simulink model "v01_aa_rii_16r4t11f_ver122sa"  (Simulink 7.6)
settings: Solver=ode45  StartTime=0.0  StopTime=128000  RelTol=1e-3
undefined symbols (not defined in the file or in its folder): sfp
libraries: xbsIndex_r4 x27287, monroe_library x154, xbsReplacements_r4 x78, casper_library_misc x33, simulink x32, dspsigops x32, xps_library x24
(part 23 of 178)

== system v01_aa_rii_16r4t11f_ver122sa/pfb_fir_real2/coeff_gen_3_12 ==
  sync : Inport
  Counter0 : xbsIndex_r4/Counter  cnt_type=Free Running  cnt_to=Inf  operation=Up  start_count=8  cnt_by_val=1  arith_type=Unsigned  n_bits=7  bin_pt=0  load_pin=off  rst=on  en=off  explicit_period=on  period=1  dbl_ovrd=off  use_behavioral_HDL=off  implementation=Fabric  block_type=counter
  Counter1 : xbsIndex_r4/Counter  cnt_type=Free Running  cnt_to=Inf  operation=Down  start_count=119  cnt_by_val=1  arith_type=Unsigned  n_bits=7  bin_pt=0  load_pin=off  rst=on  en=off  explicit_period=on  period=1  dbl_ovrd=off  use_behavioral_HDL=off  implementation=Fabric  block_type=counter
  Delay16 : xbsIndex_r4/Delay  rst=off  infoeditControl=Selection of Reset will increase slice count due to use of real FFs and instead of SRLs  en=off  latency=2  dbl_ovrd=off  reg_retiming=off  block_type=delay
  Delay17 : xbsIndex_r4/Delay  rst=off  infoeditControl=Selection of Reset will increase slice count due to use of real FFs and instead of SRLs  en=off  latency=1  dbl_ovrd=off  reg_retiming=off  block_type=delay
  Delay18 : xbsIndex_r4/Delay  rst=off  infoeditControl=Selection of Reset will increase slice count due to use of real FFs and instead of SRLs  en=off  latency=1  dbl_ovrd=off  reg_retiming=off  block_type=delay
  Delay19 : xbsIndex_r4/Delay  rst=off  infoeditControl=Selection of Reset will increase slice count due to use of real FFs and instead of SRLs  en=off  latency=2  dbl_ovrd=off  reg_retiming=off  block_type=delay
  coeff_ram_1 : xbsIndex_r4/Dual Port RAM  depth=128  initVector=[-6.84185504899742e-05 -0.000382857821943778 -0.000700524248323099 -0.00102208509462138 -0.00134821437640948 -0.00167959124907416 -0.00201689836785779 -0.00236082022082727 -0.00271204143695053 -0.00307124507150638 -0.00343911087109885 -0.00381631352058928 -0.00420352087429949 -0.00460139217387592 -0.00501057625523885 -0.00543170974707191 -0.0058654152633352 -0.00631229959231075 -0.00677295188471086 -0.00724794184339896 -0.00773781791728902 -0.00824310550200131 -0.00876430514986291 -0.00930189079184692 -0.00985630797404788 -0.0104279721112905 -0.0110172667604652 -0.0116245419161785 -0.0122501123312931 -0.0128942558649243 -0.0135572118604367 -0.0142391795559714 -0.0149403165300052 -0.0156607371844211 -0.0164005112675375 -0.0171596624395113 -0.0179381668824935 -0.0187359519578779 -0.0195528949129399 -0.0203888216391169 -0.0212435054841348 -0.0221166661201311 -0.0230079684698733 -0.0239170216931123 -0.0248433782350494 -0.0257865329388359 -0.0267459222239541 -0.0277209233322634 -0.0287108536434236 -0.029714970061332 -0.0307324684731381 -0.0317624832823181 -0.0328040870172151 -0.0338562900163623 -0.0349180401918279 -0.0359882228717288 -0.0370656607229749 -0.038149113755212 -0.0392372794068422 -0.0403287927139051 -0.0414222265625081 -0.0425160920253971 -0.0436088387831623 -0.0446988556304727 -0.0457844710676339 -0.0468639539776635 -0.0479355143889729 -0.0489973043236477 -0.050047418731209 -0.0510838965076389 -0.0521047215993477 -0.0531078241916569 -0.0540910819812655 -0.0550523215320667 -0.0559893197135738 -0.0568998052211139 -0.0577814601768411 -0.0586319218105215 -0.059448784218937 -0.0602296002026545 -0.0609718831788084 -0.0616731091684402 -0.0623307188568461 -0.0629421197252817 -0.0635046882522795 -0.0640157721827404 -0.0644726928628674 -0.0648727476389184 -0.0652132123176663 -0.0654913436863688 -0.0657043820899625 -0.0658495540631149 -0.0659240750146882 -0.0659251519620883 -0.0658499863128975 -0.0656957766911188 -0.0654597218052851 -0.0651390233556215 -0.0647308889773858 -0.0642325352174459 -0.0636411905410978 -0.0629540983660725 -0.0621685201206242 -0.0612817383225471 -0.0602910596759198 -0.0591938181823348 -0.0579873782633317 -0.0566691378907179 -0.055236531721427 -0.0536870342335396 -0.0520181628600644 -0.0502274811170587 -0.0483126017226495 -0.0462711897035023 -0.0441009654852791 -0.0417997079636163 -0.0393652575521568 -0.0367955192041694 -0.0340884654042976 -0.0312421391269863 -0.028254656758155 -0.0251242109766973 -0.021849073592414 -0.0184275983370102 -0.0148582236048162 -0.0111394751399288 -0.00726996866650266 -0.00324841245896634]  distributed_mem=Block RAM  init_a=0  init_b=0  rst_a=off  rst_b=off  en_a=off  en_b=off  latency=2  write_mode_A=No Read On Write  write_mode_B=No Read On Write  dbl_ovrd=off  optimize=Area  block_type=dpram
  coeff_ram_2 : xbsIndex_r4/Dual Port RAM  depth=128  initVector=[0.000926390151018473 0.00525553833398869 0.00974003139158092 0.0143807664579271 0.0191785362765084 0.0241340270553677 0.029247816403515 0.0345203713512892 0.0399520464573652 0.0455430820050192 0.0512936022901863 0.0572036140037557 0.0632730047104714 0.0695015414267057 0.0758888692992913 0.0824345103874953 0.0891378625501222 0.0959981984396374 0.103014664605091 0.110186280705523 0.117511938835429 0.124990402963731 0.132620308487624 0.140400161902524 0.148328340589239 0.156403092719369 0.164622537279825 0.172984664217222 0.181487334702801 0.190128281518395 0.198905109563833 0.20781529648607 0.216856193430172 0.226025025912192 0.235318894813825 0.244734777498616 0.254269529049357 0.263919883626188 0.273682455944801 0.283553742873988 0.293530125151685 0.303607869218511 0.313783129167691 0.324051948810107 0.334410263853137 0.344853904191773 0.355378596310409 0.36597996579359 0.376653539943846 0.387394750504671 0.398198936486543 0.409061347093818 0.419977144750163 0.430941408220135 0.441949135824379 0.452995248745804 0.464074594424031 0.475181950035267 0.486312026054677 0.497459469898254 0.508618869641048 0.519784757808577 0.530951615238117 0.54211387500652 0.553265926421101 0.564402119070086 0.575516766929012 0.586604152519439 0.597658531116231 0.608674134999635 0.619645177748309 0.6305658585694 0.641430366661742 0.652232885608173 0.662967597792947 0.673628688840178 0.684210352069207 0.694706792962786 0.705112233643903 0.7154209173571 0.725627112950088 0.735725119351461 0.745709270040315 0.755573937503563 0.765313537676731 0.774922534364069 0.784395443633751 0.793726838184032 0.802911351676187 0.811943683030113 0.820818600678503 0.829530946775514 0.838075641355911 0.846447686440694 0.854642170085277 0.862654270366307 0.870479259303306 0.87811250671135 0.885549483981054 0.892785767782227 0.899817043687609 0.906639109713177 0.913247879771583 0.919639387035394 0.925809787206842 0.931755361690913 0.937472520668701 0.942957806068012 0.948207894428338 0.953219599657401 0.957989875676573 0.962515818952598 0.966794670913127 0.970823820243721 0.974600805064058 0.978123314981223 0.981389193018064 0.984396437414738 0.98714320330165 0.989627804242188 0.991848713643696 0.993804566035335 0.995494158211552 0.996916450240055 0.998070566333287 0.998955795582552 0.999571592554069 0.999917577746371]  distributed_mem=Block RAM  init_a=0  init_b=0  rst_a=off  rst_b=off  en_a=off  en_b=off  latency=2  write_mode_A=No Read On Write  write_mode_B=No Read On Write  dbl_ovrd=off  optimize=Area  block_type=dpram
  coeff_ram_3 : xbsIndex_r4/Dual Port RAM  depth=128  initVector=[0.999993537908591 0.999799426219336 0.99933536232596 0.998601632244202 0.997598688118291 0.996327147841752 0.994787794539281 0.992981575910205 0.990909603434169 0.988573151439829 0.985973656037472 0.983112713916599 0.97999208100967 0.976613671023305 0.972979553838393 0.969091953780674 0.964953247763494 0.960565963304546 0.955932776418553 0.951056509387942 0.945940128413698 0.940586741148704 0.934999594115963 0.929182070014235 0.92313768491372 0.916870085344521 0.910383045280722 0.903680463023032 0.896766357983015 0.889644867372044 0.882320242798193 0.874796846774378 0.867079149141119 0.859171723407407 0.851079243013208 0.842806477517219 0.834358288713558 0.825739626681142 0.81695552576954 0.808011100525185 0.798911541561843 0.789662111379308 0.780268140134329 0.77073502136781 0.761068207692364 0.751273206444335 0.74135557530442 0.731320917891057 0.721174879330752 0.710923141809532 0.700571420109737 0.690125457136339 0.679591019437003 0.668973892720087 0.658279877374778 0.647514783997532 0.636684428928989 0.625794629805507 0.614851201129411 0.603859949862066 0.592826671043806 0.581757143444736 0.570657125250384 0.559532349786106 0.548388521284143 0.537231310697124 0.526066351561788 0.51489923591661 0.503735510276982 0.492580671671482 0.48144016374275 0.470319372916355 0.459223624641001 0.448158179703323 0.437128230620413 0.426138898113178 0.415195227663486 0.404302186157994 0.393464658621442 0.382687445042102 0.371975257291953 0.361332716144072 0.350764348389594 0.340274584056508 0.329867753732433 0.319548085993394 0.309319704940528 0.299186627846502 0.289152762913333 0.279221907143152 0.269397744323361 0.259683843127481 0.250083655332892 0.240600514156511 0.231237632709359 0.221998102570821 0.212884892483285 0.20390084716771 0.195048686260562 0.186331003372418 0.177750265268411 0.169308811170563 0.161008852181946 0.152852470832436 0.144841620745766 0.136978126427406 0.129263683172704 0.121699857094577 0.114288085269957 0.107029676004035 0.0999258092112481 0.092977536911857 0.0861857838428 0.0795513481814435 0.0730749023807116 0.0667569941139751 0.0605980473279757 0.0545983634019544 0.0487581224110534 0.0430773844919589 0.0375560913086581 0.0321940676160892 0.0269910229193702 0.0219465532262078 0.0170601428899978 0.0123311665410521 0.00775889110330314 0.00334247789376452]  distributed_mem=Block RAM  init_a=0  init_b=0  rst_a=off  rst_b=off  en_a=off  en_b=off  latency=2  write_mode_A=No Read On Write  write_mode_B=No Read On Write  dbl_ovrd=off  optimize=Area  block_type=dpram
  coeff_ram_4 : xbsIndex_r4/Dual Port RAM  depth=128  initVector=[-0.000919015198046551 -0.00502663145358993 -0.0089815129946132 -0.0127848983812844 -0.0164381201421957 -0.0199426022393407 -0.023299857471221 -0.0265114848172841 -0.029579166726936 -0.0325046663564158 -0.0352898247568515 -0.0379365580168502 -0.0404468543630039 -0.0428227712217146 -0.045066432245766 -0.0471800243090809 -0.0491657944731203 -0.0510260469283856 -0.052763139914492 -0.0543794826222791 -0.0558775320814249 -0.05725979003702 -0.0585287998185482 -0.0596871432047058 -0.060737437287472 -0.0616823313388219 -0.0625245036834464 -0.0632666585808146 -0.0639115231198798 -0.0644618441296943 -0.0649203851091579 -0.0652899231790798 -0.0655732460596878 -0.0657731490766683 -0.0658924321987641 -0.0659338971099046 -0.0659003443187784 -0.0657945703086977 -0.0656193647305391 -0.0653775076414712 -0.0650717667921157 -0.0647048949647076 -0.0642796273647465 -0.063798679068552 -0.0632647425290539 -0.0626804851420626 -0.0620485468751832 -0.0613715379614439 -0.0606520366596237 -0.0598925870831692 -0.0590956970995 -0.0582638363014045 -0.0573994340521345 -0.0565048776057046 -0.0555825103038107 -0.0546346298506727 -0.0536634866670147 -0.0526712823242868 -0.0516601680601348 -0.0506322433760179 -0.049589554717773 -0.0485340942398192 -0.0474677986535914 -0.0463925481606885 -0.0453101654711176 -0.04422241490691 -0.0431310015912819 -0.0420375707234105 -0.0409437069387892 -0.0398509337550292 -0.0387607131028666 -0.0376744449420393 -0.036593466961594 -0.0355190543640875 -0.0344524197330471 -0.0333947129829612 -0.0323470213909745 -0.0313103697093688 -0.0302857203578219 -0.0292739736943434 -0.0282759683637013 -0.0272924817220655 -0.0263242303365116 -0.025371870557947 -0.0244359991659379 -0.0235171540838455 -0.0226158151625978 -0.0217324050313552 -0.0208672900132567 -0.0200207811043675 -0.0191931350138819 -0.0183845552635757 -0.0175951933444434 -0.016825149928397 -0.0160744761328528 -0.0153431748359811 -0.0146312020403452 -0.013938468282616 -0.0132648400870021 -0.0126101414600018 -0.0119741554240467 -0.0113566255875756 -0.0107572577490491 -0.01017572153239 -0.00961165205131369 -0.00906465159999327 -0.00853429136749012 -0.00802011317336735 -0.00752163122189643 -0.00703833387226098 -0.00656968542216025 -0.00611512790221581 -0.00567408287858967 -0.00524595326122963 -0.00483012511516859 -0.00442596947231902 -0.00403284414122052 -0.00365009551221927 -0.00327706035558136 -0.00291306761006871 -0.00255744015953536 -0.00220949659513491 -0.00186855296076468 -0.00153392447941061 -0.00120492725809752 -0.000880879969193398 -0.000561105505862094 -0.000244932609507906]  distributed_mem=Block RAM  init_a=0  init_b=0  rst_a=off  rst_b=off  en_a=off  en_b=off  latency=2  write_mode_A=No Read On Write  write_mode_B=No Read On Write  dbl_ovrd=off  optimize=Area  block_type=dpram
  const_din : xbsIndex_r4/Constant  const=0  gui_display_data_type=Fixed-point  arith_type=Signed (2's comp)  n_bits=18  bin_pt=17  preci_type=Single  exp_width=8  frac_width=24  explicit_period=on  period=1  equ=P=C  opselect=C  inp2=PCIN>>17  opr=+  inp1=P  carry=CIN  dbl_ovrd=off  block_type=constant
  const_we : xbsIndex_r4/Constant  const=0  gui_display_data_type=Fixed-point  arith_type=Boolean  n_bits=16  bin_pt=14  preci_type=Single  exp_width=8  frac_width=24  explicit_period=on  period=1  equ=P=C  opselect=C  inp2=PCIN>>17  opr=+  inp1=P  carry=CIN  dbl_ovrd=off  block_type=constant
  cram_lower : Subsystem
  cram_upper : Subsystem
  sync_out : Outport
  coeff : Outport  Port=2
  coeff_rev : Outport  Port=3
  wires:
  sync/1 -> Counter1/1, Counter0/1, sync_out/1
  Counter0/1 -> coeff_ram_4/1, coeff_ram_3/1, Delay17/1, Delay16/1
  const_din/1 -> coeff_ram_4/5, coeff_ram_4/2, coeff_ram_3/5, coeff_ram_3/2, coeff_ram_2/5, coeff_ram_2/2, coeff_ram_1/5, coeff_ram_1/2
  const_we/1 -> coeff_ram_4/6, coeff_ram_4/3, coeff_ram_3/6, coeff_ram_3/3, coeff_ram_2/6, coeff_ram_2/3, coeff_ram_1/6, coeff_ram_1/3
  Counter1/1 -> Delay19/1, Delay18/1, coeff_ram_2/4, coeff_ram_1/4
  cram_lower/1(out) -> coeff_rev/1
  cram_upper/1(out) -> coeff/1
  coeff_ram_1/2 -> cram_lower/4(in3)
  coeff_ram_2/2 -> cram_lower/3(in2)
  coeff_ram_3/2 -> cram_lower/2(in1)
  coeff_ram_4/2 -> cram_lower/1(in0)
  coeff_ram_4/1 -> cram_upper/4(in3)
  coeff_ram_3/1 -> cram_upper/3(in2)
  coeff_ram_2/1 -> cram_upper/2(in1)
  coeff_ram_1/1 -> cram_upper/1(in0)
  Delay19/1 -> coeff_ram_4/4
  Delay18/1 -> coeff_ram_3/4
  Delay17/1 -> coeff_ram_2/1
  Delay16/1 -> coeff_ram_1/1

== system v01_aa_rii_16r4t11f_ver122sa/pfb_fir_real2/coeff_gen_4_11 ==
  sync : Inport
  Counter0 : xbsIndex_r4/Counter  cnt_type=Free Running  cnt_to=Inf  operation=Up  start_count=8  cnt_by_val=1  arith_type=Unsigned  n_bits=7  bin_pt=0  load_pin=off  rst=on  en=off  explicit_period=on  period=1  dbl_ovrd=off  use_behavioral_HDL=off  implementation=Fabric  block_type=counter
  Counter1 : xbsIndex_r4/Counter  cnt_type=Free Running  cnt_to=Inf  operation=Down  start_count=119  cnt_by_val=1  arith_type=Unsigned  n_bits=7  bin_pt=0  load_pin=off  rst=on  en=off  explicit_period=on  period=1  dbl_ovrd=off  use_behavioral_HDL=off  implementation=Fabric  block_type=counter
  Delay21 : xbsIndex_r4/Delay  rst=off  infoeditControl=Selection of Reset will increase slice count due to use of real FFs and instead of SRLs  en=off  latency=2  dbl_ovrd=off  reg_retiming=off  block_type=delay
  Delay22 : xbsIndex_r4/Delay  rst=off  infoeditControl=Selection of Reset will increase slice count due to use of real FFs and instead of SRLs  en=off  latency=1  dbl_ovrd=off  reg_retiming=off  block_type=delay
  Delay23 : xbsIndex_r4/Delay  rst=off  infoeditControl=Selection of Reset will increase slice count due to use of real FFs and instead of SRLs  en=off  latency=1  dbl_ovrd=off  reg_retiming=off  block_type=delay
  Delay24 : xbsIndex_r4/Delay  rst=off  infoeditControl=Selection of Reset will increase slice count due to use of real FFs and instead of SRLs  en=off  latency=2  dbl_ovrd=off  reg_retiming=off  block_type=delay
  coeff_ram_1 : xbsIndex_r4/Dual Port RAM  depth=128  initVector=[-8.79889735083741e-05 -0.000402610543136231 -0.000720500743932349 -0.00104232730941007 -0.00136876462388255 -0.00170049210981491 -0.00203819258610918 -0.00238255059945312 -0.00273425073091437 -0.00309397588000857 -0.0034624055285153 -0.00384021398635792 -0.00422806862190256 -0.00462662807906873 -0.0050365404836776 -0.00545844164149491 -0.0058929532304537 -0.00634068098956678 -0.00680221290706094 -0.00727811741028381 -0.00776894155994971 -0.00827520925130363 -0.00879741942479189 -0.00933604428883371 -0.00989152755729142 -0.0104642827042361 -0.0110546912386024 -0.0116631010013185 -0.0122898244874881 -0.0129351371961867 -0.0135992760104181 -0.0142824376097579 -0.0149847769181846 -0.0157064055895765 -0.0164473905333187 -0.0172077524824336 -0.0179874646066126 -0.0187864511724837 -0.0196045862534134 -0.0204416924910876 -0.0212975399110761 -0.0221718447945254 -0.0230642686080765 -0.0239744169940429 -0.0249018388228245 -0.0258460253094707 -0.0268064091962394 -0.0277823640029301 -0.0287732033466972 -0.0297781803329794 -0.0307964870190998 -0.0318272539520174 -0.0328695497816274 -0.0339223809509266 -0.0349846914642725 -0.0360553627348824 -0.0371332135126239 -0.0382169998930642 -0.0393054154086462 -0.0403970912027733 -0.0414905962874812 -0.0425844378852857 -0.0436770618556918 -0.0447668532067534 -0.0458521366919714 -0.0469311774927178 -0.0480021819862691 -0.0490632985994332 -0.0501126187476476 -0.0511481778593241 -0.0521679564851118 -0.0531698814916457 -0.0541518273392423 -0.0551116174429017 -0.0560470256158701 -0.0569557775949133 -0.0578355526463479 -0.058683985251774 -0.0594986668723528 -0.060277147790367 -0.0610169390267068 -0.0617155143328191 -0.062370312255564 -0.0629787382733223 -0.0635381670016044 -0.0640459444663137 -0.0644993904427296 -0.0648958008581796 -0.0652324502562844 -0.0655065943205716 -0.0657154724551687 -0.0658563104202036 -0.0659263230194608 -0.0659227168377624 -0.0658426930254688 -0.0656834501274199 -0.0654421869535685 -0.0651161054884889 -0.0647024138368813 -0.064198329202127 -0.0636010808948972 -0.0629079133687547 -0.0621160892796445 -0.0612228925661118 -0.0602256315470477 -0.0591216420337153 -0.0579082904527735 -0.0565829769769793 -0.0551431386602185 -0.0535862525734862 -0.0519098389384146 -0.0501114642549257 -0.0481887444195689 -0.046139347831091 -0.043960998479778 -0.0416514790171007 -0.039208633802197 -0.0366303719217263 -0.0339146701796359 -0.0310595760533919 -0.0280632106132407 -0.0249237714010852 -0.0216395352655827 -0.0182088611500971 -0.0146301928301678 -0.0109020615971933 -0.00702308888506142 -0.00299198883650501]  distributed_mem=Block RAM  init_a=0  init_b=0  rst_a=off  rst_b=off  en_a=off  en_b=off  latency=2  write_mode_A=No Read On Write  write_mode_B=No Read On Write  dbl_ovrd=off  optimize=Area  block_type=dpram
  coeff_ram_2 : xbsIndex_r4/Dual Port RAM  depth=128  initVector=[0.00119242919399737 0.00553125820390502 0.0100254911759591 0.0146760187842086 0.0194836271756769 0.0244489958305669 0.0295726955038333 0.0348551862508816 0.040296815540078 0.0458978164546789 0.0516583059867068 0.057578283425216 0.0636576288413061 0.0698961016721493 0.0762933394062088 0.0828488563717259 0.089562042630458 0.0964321629785505 0.103458356056317 0.110639633568606 0.117974879617314 0.125462850147503 0.133102172508466 0.140891345130968 0.148828737321782 0.156912589176507 0.165141011611557 0.173511986516071 0.182023367024387 0.190672877909583 0.199458116098491 0.208376551308429 0.217425526805815 0.22660226028665 0.235903844878785 0.245327250265711 0.254869323931514 0.264526792526511 0.274296263352921 0.284174225969862 0.294157053916756 0.304241006554183 0.314422231021032 0.324696764306712 0.335060535437048 0.345509367772363 0.356038981416135 0.366644995732486 0.377322931970653 0.388068215994463 0.398876181114731 0.409742071022372 0.420661042819923 0.431628170149044 0.44263844641148 0.453686788080837 0.464768038102454 0.475876969378513 0.487008288335481 0.498156638570832 0.509316604575952 0.520482715532013 0.53164944917552 0.542811235730172 0.553962461901578 0.565097474931304 0.576210586706653 0.587296077922522 0.598348202291596 0.609361190799097 0.620329255998239 0.631246596342501 0.642107400550756 0.652905852001274 0.663636133150573 0.674292429973034 0.6848689364172 0.695359858874611 0.705759420657041 0.716061866477951 0.726261466933986 0.736352522982309 0.746329370409569 0.756186384288308 0.765917983416591 0.775518634736677 0.78498285772853 0.794305228774019 0.803480385487643 0.812503031009668 0.821367938257571 0.830069954131732 0.83860400367135 0.846965094156595 0.855148319153063 0.863148862494636 0.870962002200922 0.878583114325501 0.886007676731241 0.893231272789072 0.900249594996598 0.907058448513082 0.913653754607345 0.92003155401525 0.926188010203508 0.932119412536625 0.937822179343916 0.943292860883595 0.948528142201048 0.953524845878516 0.958279934673478 0.962790514043185 0.967053834552856 0.971067294165192 0.974828440408965 0.978334972424558 0.981584742884447 0.984575759786759 0.987306188120125 0.989774351398217 0.991978733062443 0.993917977751445 0.99559089243613 0.996996447419143 0.998133777197775 0.999002181189487 0.999601124319308 0.999930237468555]  distributed_mem=Block RAM  init_a=0  init_b=0  rst_a=off  rst_b=off  en_a=off  en_b=off  latency=2  write_mode_A=No Read On Write  write_mode_B=No Read On Write  dbl_ovrd=off  optimize=Area  block_type=dpram
  coeff_ram_3 : xbsIndex_r4/Dual Port RAM  depth=128  initVector=[0.999989317784411 0.999778328850077 0.999297400715319 0.998546829787384 0.997527078582383 0.996238775337408 0.994682713483737 0.992859850981665 0.9907713095176 0.988418373564228 0.985802489304657 0.982925263421605 0.979788461752817 0.976394007814026 0.972743981190928 0.968840615801715 0.964686298031906 0.960283564743274 0.955635101158839 0.950743738625992 0.945612452259933 0.940244358469748 0.934642712369514 0.928810905076992 0.922752460902521 0.916471034430877 0.909970407498925 0.903254486072019 0.896327297022184 0.889192984811226 0.881855808081977 0.874320136160995 0.866590445476112 0.858671315892297 0.850567426969378 0.842283554145252 0.833824564848259 0.825195414542469 0.816401142709704 0.807446868772144 0.798337787959446 0.789079167124339 0.779676340510692 0.770134705478122 0.760459718187202 0.750656889249406 0.740731779345898 0.730689994819365 0.720537183243032 0.710279028971086 0.699921248674682 0.68946958686775 0.678929811426806 0.668307709108963 0.657609081072333 0.646839738403009 0.636005497652767 0.625112176391646 0.614165588779514 0.603171541160694 0.592135827685706 0.581064225964134 0.569962492752582 0.558836359681638 0.547691529025715 0.536533669519589 0.525368412225376 0.51420134645366 0.503038015742378 0.491883913897031 0.480744481095709 0.469625100062317 0.458531092311357 0.447467714467479 0.43644015466298 0.425453529016308 0.414512878194538 0.403623164062721 0.392789266422851 0.382015979845165 0.37130801059432 0.36066997365294 0.350106389844876 0.339621683060439 0.32922017758574 0.318906095538162 0.308683554409866 0.298556564721129 0.288529027785176 0.27860473358605 0.268787358770969 0.259080464758442 0.249487495963348 0.240011778140024 0.230656516844288 0.221424796015206 0.212319576677266 0.203343695763531 0.194499865060165 0.18579067027265 0.17721857021385 0.168785896113961 0.160494851052275 0.152347509510534 0.144345817047543 0.136491590094586 0.128786515871061 0.121232152419619 0.113829928759999 0.106581145160602 0.0994869735267415 0.0925484579043931 0.0857665150981551 0.0791419354020027 0.0726753834413258 0.066367399124622 0.0602183987031135 0.0542286759364502 0.0483984033625621 0.0427276336696222 0.037216301167989 0.0318642233598974 0.0266711026045835 0.0216365278764349 0.0167599766136753 0.0120408166550125 0.00747830826159482 0.00307160622155272]  distributed_mem=Block RAM  init_a=0  init_b=0  rst_a=off  rst_b=off  en_a=off  en_b=off  latency=2  write_mode_A=No Read On Write  write_mode_B=No Read On Write  dbl_ovrd=off  optimize=Area  block_type=dpram
  coeff_ram_4 : xbsIndex_r4/Dual Port RAM  depth=128  initVector=[-0.00118023796567625 -0.00527827382810551 -0.00922364958597333 -0.013017609745528 -0.0166614926248753 -0.0201567278149964 -0.0235048335790933 -0.0267074141934669 -0.0297661572331744 -0.0326828308057554 -0.0354592807363472 -0.0380974277075456 -0.0405992643573931 -0.0429668523389002 -0.0452023193445276 -0.0473078560990698 -0.0492857133243973 -0.0511381986795172 -0.0528676736794223 -0.0544765505961947 -0.0559672893458285 -0.0573423943642283 -0.0586044114758296 -0.0597559247582714 -0.0607995534065326 -0.0617379485999223 -0.0625737903752864 -0.0633097845097633 -0.0639486594163894 -0.0644931630558156 -0.0649460598673577 -0.0653101277225574 -0.0655881549043853 -0.0657829371151641 -0.0658972745162394 -0.0659339688023646 -0.0658958203137091 -0.0657856251883347 -0.0656061725579181 -0.0653602417894302 -0.0650505997754095 -0.0646799982753933 -0.0642511713109933 -0.0637668326170237 -0.0632296731510054 -0.0626423586632902 -0.0620075273299594 -0.0613277874505636 -0.060605715212683 -0.0598438525251917 -0.0590447049220196 -0.0582107395381091 -0.0573443831591655 -0.0564480203467063 -0.0555239916398113 -0.0545745918348781 -0.0536020683445854 -0.0526086196371647 -0.0515963937569784 -0.0505674869273002 -0.0495239422360871 -0.0484677484054324 -0.0474008386452801 -0.0463250895918826 -0.0452423203313728 -0.0441542915087227 -0.0430627045222547 -0.0419692008037677 -0.0408753611842385 -0.0397827053449559 -0.0386926913538438 -0.0376067152866287 -0.0365261109324065 -0.0354521495830675 -0.0343860399059371 -0.0333289278988981 -0.0322818969271628 -0.0312459678407708 -0.0302220991717978 -0.0292111874101705 -0.0282140673568951 -0.02723151255342 -0.0262642357857716 -0.0253128896620191 -0.0243780672615441 -0.0234603028545157 -0.022560072689895 -0.0216777958502221 -0.0208138351713685 -0.0199684982253701 -0.0191420383643933 -0.0183346558238221 -0.0175464988823994 -0.0167776650772961 -0.0160282024719291 -0.0152981109743031 -0.0145873437035975 -0.0138958084026824 -0.0132233688942021 -0.0125698465778293 -0.0119350219662582 -0.0113186362574725 -0.0107203929407984 -0.0101399594342249 -0.00957696875045512 -0.00903102118913259 -0.00850168605267101 -0.00798850338310597 -0.00749098571737739 -0.00700861985844697 -0.00654086865965289 -0.00608717281970556 -0.00564695268573309 -0.00521961006179269 -0.00480453002027584 -0.00440108271364903 -0.00400862518398944 -0.00362650316779578 -0.00325405289357752 -0.00289060286975338 -0.00253547566041853 -0.0021879896465735 -0.00184746077044269 -0.00151320426054887 -0.00118453633525111 -0.000860775882497438 -0.00054124611358964 -0.000225276188806986]  distributed_mem=Block RAM  init_a=0  init_b=0  rst_a=off  rst_b=off  en_a=off  en_b=off  latency=2  write_mode_A=No Read On Write  write_mode_B=No Read On Write  dbl_ovrd=off  optimize=Area  block_type=dpram
  const_din : xbsIndex_r4/Constant  const=0  gui_display_data_type=Fixed-point  arith_type=Signed (2's comp)  n_bits=18  bin_pt=17  preci_type=Single  exp_width=8  frac_width=24  explicit_period=on  period=1  equ=P=C  opselect=C  inp2=PCIN>>17  opr=+  inp1=P  carry=CIN  dbl_ovrd=off  block_type=constant
  const_we : xbsIndex_r4/Constant  const=0  gui_display_data_type=Fixed-point  arith_type=Boolean  n_bits=16  bin_pt=14  preci_type=Single  exp_width=8  frac_width=24  explicit_period=on  period=1  equ=P=C  opselect=C  inp2=PCIN>>17  opr=+  inp1=P  carry=CIN  dbl_ovrd=off  block_type=constant
  cram_lower : Subsystem
  cram_upper : Subsystem
  sync_out : Outport
  coeff : Outport  Port=2
  coeff_rev : Outport  Port=3
  wires:
  sync/1 -> Counter1/1, Counter0/1, sync_out/1
  Counter0/1 -> coeff_ram_4/1, coeff_ram_3/1, Delay22/1, Delay21/1
  const_din/1 -> coeff_ram_4/5, coeff_ram_4/2, coeff_ram_3/5, coeff_ram_3/2, coeff_ram_2/5, coeff_ram_2/2, coeff_ram_1/5, coeff_ram_1/2
  const_we/1 -> coeff_ram_4/6, coeff_ram_4/3, coeff_ram_3/6, coeff_ram_3/3, coeff_ram_2/6, coeff_ram_2/3, coeff_ram_1/6, coeff_ram_1/3
  Counter1/1 -> Delay24/1, Delay23/1, coeff_ram_2/4, coeff_ram_1/4
  cram_lower/1(out) -> coeff_rev/1
  cram_upper/1(out) -> coeff/1
  coeff_ram_1/2 -> cram_lower/4(in3)
  coeff_ram_2/2 -> cram_lower/3(in2)
  coeff_ram_3/2 -> cram_lower/2(in1)
  coeff_ram_4/2 -> cram_lower/1(in0)
  coeff_ram_4/1 -> cram_upper/4(in3)
  coeff_ram_3/1 -> cram_upper/3(in2)
  coeff_ram_2/1 -> cram_upper/2(in1)
  coeff_ram_1/1 -> cram_upper/1(in0)
  Delay24/1 -> coeff_ram_4/4
  Delay23/1 -> coeff_ram_3/4
  Delay22/1 -> coeff_ram_2/1
  Delay21/1 -> coeff_ram_1/1

== system v01_aa_rii_16r4t11f_ver122sa/pfb_fir_real2/coeff_gen_5_10 ==
  sync : Inport
  Counter0 : xbsIndex_r4/Counter  cnt_type=Free Running  cnt_to=Inf  operation=Up  start_count=8  cnt_by_val=1  arith_type=Unsigned  n_bits=7  bin_pt=0  load_pin=off  rst=on  en=off  explicit_period=on  period=1  dbl_ovrd=off  use_behavioral_HDL=off  implementation=Fabric  block_type=counter
  Counter1 : xbsIndex_r4/Counter  cnt_type=Free Running  cnt_to=Inf  operation=Down  start_count=119  cnt_by_val=1  arith_type=Unsigned  n_bits=7  bin_pt=0  load_pin=off  rst=on  en=off  explicit_period=on  period=1  dbl_ovrd=off  use_behavioral_HDL=off  implementation=Fabric  block_type=counter
  Delay26 : xbsIndex_r4/Delay  rst=off  infoeditControl=Selection of Reset will increase slice count due to use of real FFs and instead of SRLs  en=off  latency=2  dbl_ovrd=off  reg_retiming=off  block_type=delay
  Delay27 : xbsIndex_r4/Delay  rst=off  infoeditControl=Selection of Reset will increase slice count due to use of real FFs and instead of SRLs  en=off  latency=1  dbl_ovrd=off  reg_retiming=off  block_type=delay
  Delay28 : xbsIndex_r4/Delay  rst=off  infoeditControl=Selection of Reset will increase slice count due to use of real FFs and instead of SRLs  en=off  latency=1  dbl_ovrd=off  reg_retiming=off  block_type=delay
  Delay29 : xbsIndex_r4/Delay  rst=off  infoeditControl=Selection of Reset will increase slice count due to use of real FFs and instead of SRLs  en=off  latency=2  dbl_ovrd=off  reg_retiming=off  block_type=delay
  coeff_ram_1 : xbsIndex_r4/Dual Port RAM  depth=128  initVector=[-0.000107569585267786 -0.000422376029748434 -0.000740492613808274 -0.00106258753330215 -0.00138933553498542 -0.00172141630198795 -0.00205951281035255 -0.00240430965877225 -0.00275649137371078 -0.00311674069213787 -0.00348573682415593 -0.00386415369783665 -0.00425265818862522 -0.004651908335707 -0.00506255154776435 -0.00548522280058271 -0.00592054282899263 -0.00636911631565927 -0.00683153007925246 -0.00730835126454939 -0.00780012553703721 -0.00830737528459525 -0.00883059782884593 -0.00937026364876885 -0.00992681461917595 -0.0105006622666443 -0.0110921860454999 -0.011701731636438 -0.0123296092703562 -0.0129760920799613 -0.0136414144816947 -0.0143257705905025 -0.0150293126699483 -0.0157521496201454 -0.0164943455059514 -0.0172559181278362 -0.0180368376377969 -0.0188370252026555 -0.019656351717031 -0.0204946365682309 -0.0213516464552603 -0.0222270942640942 -0.0231206380013017 -0.0240318797880571 -0.024960364916507 -0.0259055809704055 -0.0268669570118572 -0.0278438628359437 -0.0288356082949348 -0.0298414426937147 -0.030860554257974 -0.0318920696766433 -0.0329350537199596 -0.0339885089344771 -0.0350513754162473 -0.0361225306633048 -0.0372007895085106 -0.0382849041337087 -0.039373564166063 -0.0404653968573466 -0.0415589673468591 -0.042652779008552 -0.0437452738828431 -0.044834833193503 -0.0459197779498935 -0.0469983696347412 -0.0480688109775221 -0.049129246813435 -0.050177765027835 -0.0512123975858969 -0.052231121647172 -0.0532318607646 -0.0542124861674312 -0.0551708181274122 -0.0561046274074803 -0.0570116367921141 -0.0578895226983776 -0.0587359168665974 -0.0595484081295078 -0.0603245442585982 -0.061061833886296 -0.0617577485025197 -0.0624097245240382 -0.0630151654349739 -0.0635714439966954 -0.0640759045252461 -0.0645258652343679 -0.0649186206420846 -0.0652514440387233 -0.0655215900141629 -0.0657262970420173 -0.0658627901183743 -0.0659282834526343 -0.0659199832079138 -0.0658350902884022 -0.0656708031709908 -0.0654243207784188 -0.0650928453911163 -0.0646735855948617 -0.0641637592613036 -0.0635605965583465 -0.0628613429873378 -0.0620632624439459 -0.0611636402995691 -0.0601597865000682 -0.0590490386785773 -0.0578287652791049 -0.056496368687605 -0.0550492883671667 -0.053485003993941 -0.051801038590402 -0.0499949616525186 -0.0480643922673949 -0.0460070022179281 -0.0438205190710197 -0.0415027292458763 -0.0390514810589286 -0.0364646877419062 -0.0337403304296094 -0.0308764611139297 -0.0278712055606872 -0.0247227661858699 -0.0214294248878829 -0.0179895458324434 -0.0144015781867842 -0.0106640587998664 -0.00677561482533706 -0.00273496628401131]  distributed_mem=Block RAM  init_a=0  init_b=0  rst_a=off  rst_b=off  en_a=off  en_b=off  latency=2  write_mode_A=No Read On Write  write_mode_B=No Read On Write  dbl_ovrd=off  optimize=Area  block_type=dpram
  coeff_ram_2 : xbsIndex_r4/Dual Port RAM  depth=128  initVector=[0.00145907143729748 0.00580758515372519 0.0103115615201561 0.0149718847418897 0.0197893343611322 0.0247645831222048 0.0298981949182939 0.0351906228224975 0.0406422072058491 0.0462531739449196 0.0520236327215227 0.0579535754169587 0.0640428746031509 0.0702912821329352 0.0766984278316722 0.0832638182922552 0.0899868357754913 0.0968667372177273 0.103902653347496 0.111093587912844 0.118438417020907 0.125935888591171 0.133584621923765 0.141383107384003 0.14932970620428 0.157422650404317 0.165660042830627 0.174039857315936 0.182559938959216 0.191218004526804 0.200011642975018 0.208938316094508 0.217995359276477 0.227179982400794 0.236489270845853 0.245920186619955 0.255469569613818 0.265134138973733 0.274910494594723 0.284795118732959 0.294784377736543 0.304874523893659 0.315061697396959 0.325341928422915 0.335711139324776 0.346165146937609 0.356699664993807 0.367310306647323 0.377992587104762 0.388741926361352 0.3995536520397 0.410423002329125 0.42134512902325 0.432315100653421 0.443327905715421 0.454378455986836 0.465461589932334 0.476572076194014 0.487704617163885 0.498853852635448 0.510014363531255 0.521180675703231 0.532347263802471 0.543508555215127 0.554658934060937 0.565792745250863 0.576904298600234 0.587987872993732 0.599037720598481 0.610048071121458 0.621013136107362 0.631927113273054 0.64278419087461 0.653578552102996 0.664304379504337 0.674955859420692 0.685527186447259 0.696012567901861 0.706406228302562 0.71670241384925 0.726895396904998 0.736979480472992 0.746949002664845 0.756798341156072 0.766521917624539 0.776114202167677 0.785569717694288 0.794883044286773 0.80404882352963 0.813061762800099 0.821916639516871 0.830608305342786 0.839131690337502 0.847481807056159 0.855653754590092 0.863642722545723 0.871443994957782 0.879052954133097 0.886465084421244 0.893675975908404 0.90068132803086 0.907476953104629 0.914058779767815 0.920422856332328 0.926565354041732 0.932482570232033 0.938170931392346 0.943626996122459 0.948847457984396 0.953829148245221 0.958569038508385 0.963064243231052 0.967312022124952 0.971309782438401 0.975055081117256 0.978545626842696 0.981779281943836 0.984754064183276 0.987468148413867 0.989919868105043 0.992107716737239 0.994030349063009 0.995686582233628 0.997075396790044 0.998195937517235 0.999047514161112 0.999629602007269 0.999941842321012]  distributed_mem=Block RAM  init_a=0  init_b=0  rst_a=off  rst_b=off  en_a=off  en_b=off  latency=2  write_mode_A=No Read On Write  write_mode_B=No Read On Write  dbl_ovrd=off  optimize=Area  block_type=dpram
  coeff_ram_3 : xbsIndex_r4/Dual Port RAM  depth=128  initVector=[0.99998404264824 0.999756176976869 0.999258385758651 0.998490975791367 0.997454419961498 0.996149356847644 0.994576590185067 0.992737088191891 0.990631982757617 0.988262568494757 0.985630301654514 0.982736798907566 0.979583835991173 0.976173346223902 0.972507418889464 0.968588297491222 0.964418377879087 0.960000206250649 0.955336477028487 0.950430030615749 0.945283851032188 0.939901063432977 0.934284931512716 0.928438854797182 0.922366365825457 0.916071127225183 0.9095569286838 0.902827683818721 0.895887426949478 0.888740309774992 0.881390597959187 0.873842667628272 0.866101001783077 0.858170186629926 0.850054907833602 0.841759946696015 0.833290176264273 0.824650557371908 0.815846134617063 0.80688203228152 0.797763450194484 0.788495659545081 0.779083998647602 0.769533868663517 0.759850729284371 0.750040094379647 0.74010752761376 0.730058638036332 0.719899075649928 0.709634526959455 0.699270710507401 0.688813372399157 0.678268281822577 0.667641226566031 0.656938008539083 0.646164439300029 0.635326335594405 0.624429514908636 0.613479791042921 0.602482969707436 0.591444844145906 0.580371190790548 0.569267764952351 0.558140296550607 0.546994485885559 0.535835999457983 0.524670465839443 0.513503471596919 0.502340557275423 0.491187213442166 0.480048876795736 0.468930926343707 0.457838679651999 0.446777389169205 0.43575223862907 0.424768339534152 0.413830727723648 0.40294436002826 0.392114111014859 0.381344769823639 0.370641037100306 0.360007522025796 0.349448739445834 0.338969107102619 0.328572942970738 0.318264462699335 0.308047777162429 0.297926890119176 0.287905695985714 0.277987977720161 0.268177404822157 0.258477531448264 0.248891794644391 0.239423512696287 0.230075883599026 0.220851983646282 0.211754766140051 0.202787060221373 0.193951569822464 0.18525087274054 0.1766874198335 0.168263534337492 0.159981411306287 0.151843117172221 0.14385058942837 0.136005636431506 0.128309937325212 0.120765042082475 0.113372371666903 0.106133218311624 0.0990487459147927 0.0921199905505177 0.0853478610939116 0.0787331399588477 0.0722764839468997 0.0659784252058317 0.0598393722959009 0.053859611362127 0.0480393074105875 0.0423785056866932 0.0368771331533055 0.0315350000664618 0.0263518016463846 0.0213271198413648 0.0164604251820201 0.0117510787233527 0.00719833407194898 0.00280133949558966]  distributed_mem=Block RAM  init_a=0  init_b=0  rst_a=off  rst_b=off  en_a=off  en_b=off  latency=2  write_mode_A=No Read On Write  write_mode_B=No Read On Write  dbl_ovrd=off  optimize=Area  block_type=dpram
  coeff_ram_4 : xbsIndex_r4/Dual Port RAM  depth=128  initVector=[-0.00144085988753332 -0.00552931984286397 -0.00946519467973126 -0.0132497348407979 -0.0168842844237644 -0.0203702786384396 -0.0237092412023377 -0.0269027816780089 -0.0299525927553551 -0.0328604474822192 -0.0356281964465746 -0.0382577649136687 -0.040751149921507 -0.0431104173380844 -0.0453376988837907 -0.047435189122435 -0.0494051424243428 -0.0512498699049901 -0.0529717363426413 -0.054573157078459 -0.0560565949025491 -0.0574245569293971 -0.0586795914661417 -0.059824284877114 -0.0608612584480534 -0.0617931652533879 -0.0626226870299403 -0.0633525310603907 -0.0639854270697942 -0.0645241241384111 -0.0649713876340721 -0.0653299961672505 -0.0656027385719703 -0.0657924109156264 -0.0659018135407379 -0.0659337481416 -0.065891014878739 -0.0657764095340109 -0.0655927207091173 -0.0653427270702455 -0.065029194641465 -0.0646548741494391 -0.0642224984219347 -0.0637347798425306 -0.0631944078638471 -0.0626040465815299 -0.0619663323711427 -0.0612838715900259 -0.0605592383460962 -0.0597949723354663 -0.0589935767506698 -0.0581575162611838 -0.0572892150678432 -0.0563910550326432 -0.0554653738853278 -0.0545144635080614 -0.0535405682993803 -0.0525458836185179 -0.0515325543110957 -0.0505026733170676 -0.0494582803617038 -0.0484013607302936 -0.0473338441271439 -0.0462576036193459 -0.0451744546656777 -0.0440861542309057 -0.0429943999856465 -0.041900829591843 -0.0408070200738111 -0.0397144872747065 -0.0386246853981613 -0.0375390066347402 -0.0364587808727664 -0.0353852754929661 -0.0343196952462879 -0.0332631822141531 -0.0322168158503007 -0.0311816131032988 -0.0301585286186988 -0.029148455019725 -0.028152223265299 -0.0271706030841177 -0.0262043034834144 -0.025253973330957 -0.0243202020087535 -0.0234035201368593 -0.0225044003656085 -0.0216232582345142 -0.0207604530960201 -0.0199162891022117 -0.0190910162525368 -0.0182848315005203 -0.0174978799174008 -0.0167302559105606 -0.0159820044945683 -0.0152531226126017 -0.0145435605059747 -0.0138532231294453 -0.0131819716099429 -0.0125296247463155 -0.0118959605476621 -0.011280717807786 -0.0106835977132754 -0.0101042654826945 -0.00954235203434532 -0.00899745568004499 -0.00846914384234494 -0.00795695479261062 -0.00746039940736976 -0.00697896294033309 -0.00651210680749025 -0.00605927038268448 -0.00561987280107531 -0.00519331476790601 -0.00477898037000458 -0.00437623888746081 -0.00398444660294039 -0.0036029486061171 -0.0032310805907286 -0.00286817064178778 -0.00251354101051151 -0.00216650987456177 -0.00182639308122933 -0.00149250587122898 -0.00116416458081627 -0.000840688319979981 -0.000521400624510641 -0.000205631079795115]  distributed_mem=Block RAM  init_a=0  init_b=0  rst_a=off  rst_b=off  en_a=off  en_b=off  latency=2  write_mode_A=No Read On Write  write_mode_B=No Read On Write  dbl_ovrd=off  optimize=Area  block_type=dpram
  const_din : xbsIndex_r4/Constant  const=0  gui_display_data_type=Fixed-point  arith_type=Signed (2's comp)  n_bits=18  bin_pt=17  preci_type=Single  exp_width=8  frac_width=24  explicit_period=on  period=1  equ=P=C  opselect=C  inp2=PCIN>>17  opr=+  inp1=P  carry=CIN  dbl_ovrd=off  block_type=constant
  const_we : xbsIndex_r4/Constant  const=0  gui_display_data_type=Fixed-point  arith_type=Boolean  n_bits=16  bin_pt=14  preci_type=Single  exp_width=8  frac_width=24  explicit_period=on  period=1  equ=P=C  opselect=C  inp2=PCIN>>17  opr=+  inp1=P  carry=CIN  dbl_ovrd=off  block_type=constant
  cram_lower : Subsystem
  cram_upper : Subsystem
  sync_out : Outport
  coeff : Outport  Port=2
  coeff_rev : Outport  Port=3
  wires:
  sync/1 -> Counter1/1, Counter0/1, sync_out/1
  Counter0/1 -> coeff_ram_4/1, coeff_ram_3/1, Delay27/1, Delay26/1
  const_din/1 -> coeff_ram_4/5, coeff_ram_4/2, coeff_ram_3/5, coeff_ram_3/2, coeff_ram_2/5, coeff_ram_2/2, coeff_ram_1/5, coeff_ram_1/2
  const_we/1 -> coeff_ram_4/6, coeff_ram_4/3, coeff_ram_3/6, coeff_ram_3/3, coeff_ram_2/6, coeff_ram_2/3, coeff_ram_1/6, coeff_ram_1/3
  Counter1/1 -> Delay29/1, Delay28/1, coeff_ram_2/4, coeff_ram_1/4
  cram_lower/1(out) -> coeff_rev/1
  cram_upper/1(out) -> coeff/1
  coeff_ram_1/2 -> cram_lower/4(in3)
  coeff_ram_2/2 -> cram_lower/3(in2)
  coeff_ram_3/2 -> cram_lower/2(in1)
  coeff_ram_4/2 -> cram_lower/1(in0)
  coeff_ram_4/1 -> cram_upper/4(in3)
  coeff_ram_3/1 -> cram_upper/3(in2)
  coeff_ram_2/1 -> cram_upper/2(in1)
  coeff_ram_1/1 -> cram_upper/1(in0)
  Delay29/1 -> coeff_ram_4/4
  Delay28/1 -> coeff_ram_3/4
  Delay27/1 -> coeff_ram_2/1
  Delay26/1 -> coeff_ram_1/1
